simulink model "SyDdemo"  (Simulink 7.0)
settings: Solver=FixedStepDiscrete  StartTime=0.0  StopTime=10.0  FixedStep=0.01  RelTol=1e-3
model workspace (MAT data in the model file):
  air_esti_error : Simulink.Signal (value not decoded)
  atmo_pressure : Simulink.Signal (value not decoded)
  battery_volt : Simulink.Signal (value not decoded)
  control_pwm : Simulink.Signal (value not decoded)
  control_volt : Simulink.Signal (value not decoded)
  controller_error : Simulink.Signal (value not decoded)
  controller_error_lim : Simulink.Signal (value not decoded)
  controller_r0_out : Simulink.Signal (value not decoded)
  controller_r1_out : Simulink.Signal (value not decoded)
  controller_r2_out : Simulink.Signal (value not decoded)
  controller_r3_out : Simulink.Signal (value not decoded)
  controller_r_out : Simulink.Signal (value not decoded)
  controller_s0_out : Simulink.Signal (value not decoded)
  controller_s1_out : Simulink.Signal (value not decoded)
  controller_s2_out : Simulink.Signal (value not decoded)
  controller_s_out : Simulink.Signal (value not decoded)
  controller_s_out_prev : Simulink.Signal (value not decoded)
  controller_t0_out : Simulink.Signal (value not decoded)
  controller_t1_out : Simulink.Signal (value not decoded)
  controller_t2_out : Simulink.Signal (value not decoded)
  controller_t_out : Simulink.Signal (value not decoded)
  coolant_temp : Simulink.Signal (value not decoded)
  diag_control_ena : Simulink.Signal (value not decoded)
  diag_sp_ena : Simulink.Signal (value not decoded)
  engine_speed : Simulink.Signal (value not decoded)
  engine_status : Simulink.Signal (value not decoded)
  friction_comp_volt : Simulink.Signal (value not decoded)
  global_control_error : Simulink.Signal (value not decoded)
  pos_dmnd_raw : Simulink.Signal (value not decoded)
  pos_feedback : Simulink.Signal (value not decoded)
  pos_offset : Simulink.Signal (value not decoded)
  pos_relative : Simulink.Signal (value not decoded)
  pos_setpoint : Simulink.Signal (value not decoded)
  COR_COOLANT_TEMP_XDAT : Simulink.Parameter (value not decoded)
  COR_COOLANT_TEMP_YDAT : Simulink.Parameter (value not decoded)
  COR_ATMO_PRESSURE_XDAT : Simulink.Parameter (value not decoded)
  COR_ATMO_PRESSURE_YDAT : Simulink.Parameter (value not decoded)
  COR_AIR_ESTI_ERROR_GAIN : Simulink.Parameter (value not decoded)
  CONTROLLER_T2 : Simulink.Parameter (value not decoded)
  CONTROLLER_T1 : Simulink.Parameter (value not decoded)
  CONTROLLER_T0 : Simulink.Parameter (value not decoded)
  CONTROLLER_SETPONT_SIGN : Simulink.Parameter (value not decoded)
  CONTROLLER_R3 : Simulink.Parameter (value not decoded)
  CONTROLLER_R2 : Simulink.Parameter (value not decoded)
  CONTROLLER_R1 : Simulink.Parameter (value not decoded)
  CONTROLLER_SIGN : Simulink.Parameter (value not decoded)
  CONTROLLER_R0 : Simulink.Parameter (value not decoded)
  CONTROLLER_S2 : Simulink.Parameter (value not decoded)
  CONTROLLER_S1 : Simulink.Parameter (value not decoded)
  CONTROLLER_S0 : Simulink.Parameter (value not decoded)
  CONTROLLER_ERROR_LIM : Simulink.Parameter (value not decoded)
  FRICTION_DYN_COMP_VOLT : Simulink.Parameter (value not decoded)
  FRICTION_COMP_THR : Simulink.Parameter (value not decoded)
  FRICTION_STATIC_COMP_VOLT : Simulink.Parameter (value not decoded)
  BATTERY_COR_ENA : Simulink.Parameter (value not decoded)
  BATTERY_COR_PWM : Simulink.Parameter (value not decoded)
  DIAG_ENGINE_SPEED_MAX : Simulink.Parameter (value not decoded)
  DIAG_AIR_ESTI_ERROR_MAX : Simulink.Parameter (value not decoded)
  DIAG_POS_DMND_RAW_MIN : Simulink.Parameter (value not decoded)
  RUNNING : Simulink.Parameter (value not decoded)
  DIAG_ENGINE_SPEED_MIN : Simulink.Parameter (value not decoded)
  DIAG_COOLANT_TEMP_MAX : Simulink.Parameter (value not decoded)
  DIAG_COOLANT_TEMP_MIN : Simulink.Parameter (value not decoded)
  DIAG_ATMO_PRESSURE_MAX : Simulink.Parameter (value not decoded)
  C_GAIN : Simulink.Parameter (value not decoded)
libraries: simulink x12
settings Model: Creator=Aurelien PROST  LastModifiedBy=LeanPulse
settings Model: ModifiedHistory=|
  | <syd:extradata xmlns:syd="http://www.leanpulse.com/schemas/syd/2011/core" owner="LeanPulse" ref="SPEC0001" title="SyD Demo Document"><syd:version author="Aurélien PROST" date="10 Jan 2011" num="1.0" opt1="a.prost@leanpulse.com" opt2="">This specification has been created for demo purpose in order to highlight the main features of the LeanPulse System Document Generator (SyD).
  | 
  | (c) LeanPulse 2011</syd:version><syd:version author="Aurélien PROST" date="11 Jan 2011" num="2.0" opt1="a.prost@leanpulse.com" opt2="">You can edit this history by using the document metadata editor available in the file menu of your model.</syd:version></syd:extradata>
settings OptimizationCC: InitFltsAndDblsToZero=off
settings DebuggingCC: StrictBusMsg=ErrorLevel1

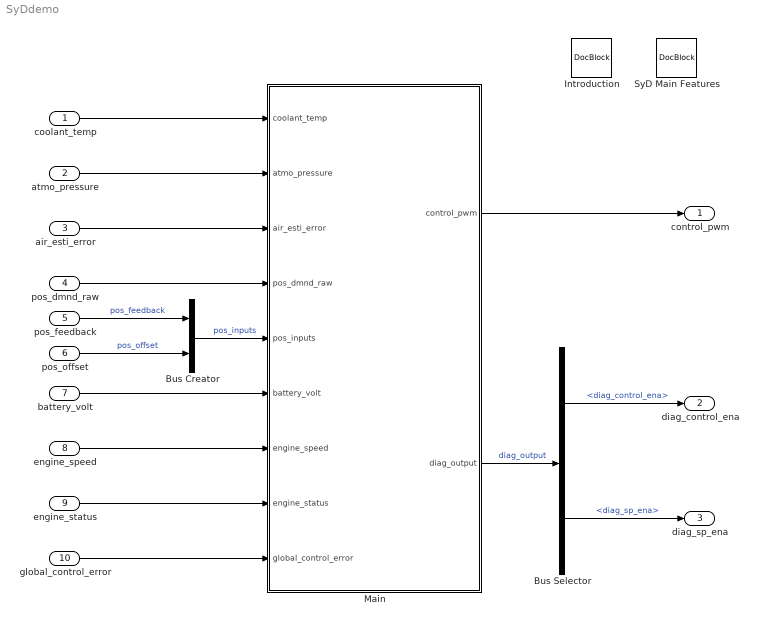
== system SyDdemo ==
  coolant_temp : Inport
  atmo_pressure : Inport  Port=2
  air_esti_error : Inport  Port=3
  pos_dmnd_raw : Inport  Port=4
  pos_feedback : Inport  Port=5
  pos_offset : Inport  Port=6
  battery_volt : Inport  Port=7
  engine_speed : Inport  Port=8
  engine_status : Inport  Port=9
  global_control_error : Inport  Port=10
  Bus Creator : BusCreator  Inputs=2
  Bus Selector : BusSelector  OutputSignals=diag_control_ena,diag_sp_ena
  Introduction : simulink/Model-Wide Utilities/DocBlock  SystemSampleTime=-1  DocumentType=RTF
  Main : Subsystem
  SyD Main Features : simulink/Model-Wide Utilities/DocBlock  SystemSampleTime=-1  DocumentType=RTF
  control_pwm : Outport
  diag_control_ena : Outport  Port=2
  diag_sp_ena : Outport  Port=3
  wires:
  coolant_temp/1 -> Main/1(coolant_temp)
  atmo_pressure/1 -> Main/2(atmo_pressure)
  air_esti_error/1 -> Main/3(air_esti_error)
  pos_dmnd_raw/1 -> Main/4(pos_dmnd_raw)
  battery_volt/1 -> Main/6(battery_volt)
  engine_speed/1 -> Main/7(engine_speed)
  engine_status/1 -> Main/8(engine_status)
  global_control_error/1 -> Main/9(global_control_error)
  Main/1(control_pwm) -> control_pwm/1
  Bus Selector/1 -> diag_control_ena/1  "<diag_control_ena>"
  Bus Selector/2 -> diag_sp_ena/1  "<diag_sp_ena>"
  pos_offset/1 -> Bus Creator/2  "pos_offset"
  pos_feedback/1 -> Bus Creator/1  "pos_feedback"
  Bus Creator/1 -> Main/5(pos_inputs)  "pos_inputs"
  Main/2(diag_output) -> Bus Selector/1  "diag_output"

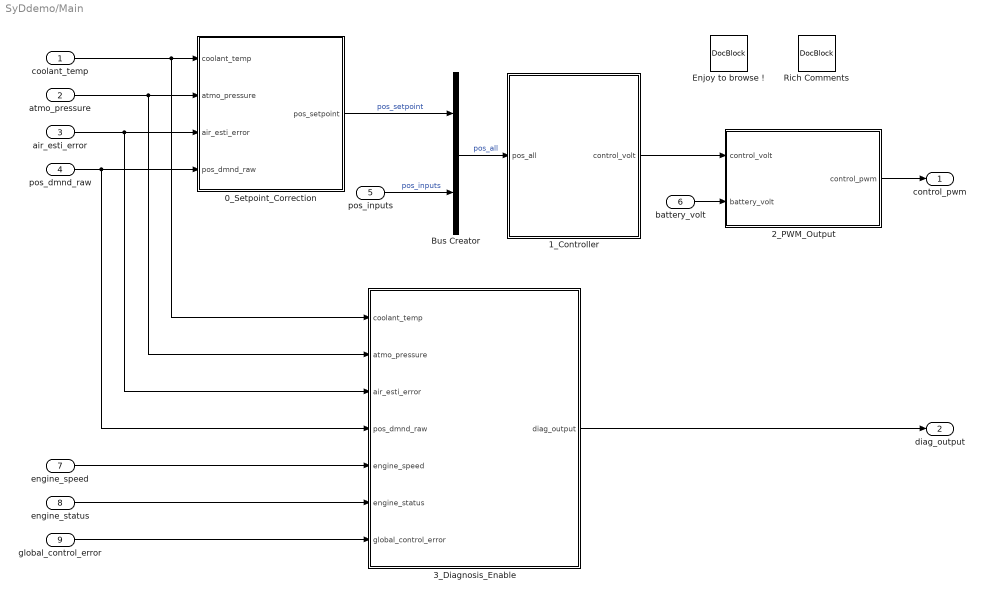
== system SyDdemo/Main ==
  coolant_temp : Inport
  atmo_pressure : Inport  Port=2
  air_esti_error : Inport  Port=3
  pos_dmnd_raw : Inport  Port=4
  pos_inputs : Inport  Port=5
  battery_volt : Inport  Port=6
  engine_speed : Inport  Port=7
  engine_status : Inport  Port=8
  global_control_error : Inport  Port=9
  0_Setpoint_Correction : Subsystem  Description="This comment has been written in the description field of the SubSystem \"0_Setpoint_Correction\"."
  1_Controller : Subsystem
  2_PWM_Output : Subsystem
  3_Diagnosis_Enable : Subsystem
  Bus Creator : BusCreator  Inputs=2
  Enjoy to browse ! : simulink/Model-Wide Utilities/DocBlock  SystemSampleTime=-1  DocumentType=RTF
  Rich Comments : simulink/Model-Wide Utilities/DocBlock  SystemSampleTime=-1  DocumentType=RTF
  control_pwm : Outport
  diag_output : Outport  Port=2
  wires:
  1_Controller/1(control_volt) -> 2_PWM_Output/1(control_volt)
  coolant_temp/1 -> 0_Setpoint_Correction/1(coolant_temp), 3_Diagnosis_Enable/1(coolant_temp)
  atmo_pressure/1 -> 0_Setpoint_Correction/2(atmo_pressure), 3_Diagnosis_Enable/2(atmo_pressure)
  air_esti_error/1 -> 0_Setpoint_Correction/3(air_esti_error), 3_Diagnosis_Enable/3(air_esti_error)
  pos_dmnd_raw/1 -> 0_Setpoint_Correction/4(pos_dmnd_raw), 3_Diagnosis_Enable/4(pos_dmnd_raw)
  battery_volt/1 -> 2_PWM_Output/2(battery_volt)
  2_PWM_Output/1(control_pwm) -> control_pwm/1
  3_Diagnosis_Enable/1(diag_output) -> diag_output/1
  0_Setpoint_Correction/1(pos_setpoint) -> Bus Creator/1  "pos_setpoint"
  pos_inputs/1 -> Bus Creator/2  "pos_inputs"
  Bus Creator/1 -> 1_Controller/1(pos_all)  "pos_all"
  engine_speed/1 -> 3_Diagnosis_Enable/5(engine_speed)
  engine_status/1 -> 3_Diagnosis_Enable/6(engine_status)
  global_control_error/1 -> 3_Diagnosis_Enable/7(global_control_error)

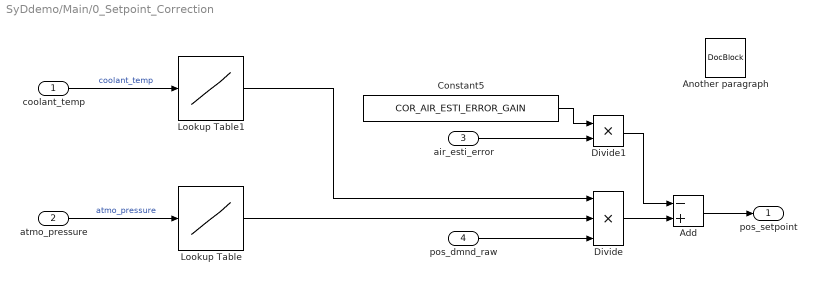
== system SyDdemo/Main/0_Setpoint_Correction ==
  coolant_temp : Inport
  atmo_pressure : Inport  Port=2
  air_esti_error : Inport  Port=3
  pos_dmnd_raw : Inport  Port=4
  Add : Sum  Inputs=-+
  Another paragraph : simulink/Model-Wide Utilities/DocBlock  SystemSampleTime=-1  DocumentType=RTF
  Constant5 : Constant  Value=COR_AIR_ESTI_ERROR_GAIN
  Divide : Product  Inputs=***
  Divide1 : Product  Inputs=**
  Lookup Table : Lookup  InputValues=COR_ATMO_PRESSURE_XDAT  Table=COR_ATMO_PRESSURE_YDAT
      settings: AttributesFormatString=Table: %<Table>\nInput Values: %<InputValues>
  Lookup Table1 : Lookup  InputValues=COR_COOLANT_TEMP_XDAT  Table=COR_COOLANT_TEMP_YDAT
      settings: AttributesFormatString=Table: %<Table>\nInput Values: %<InputValues>
  pos_setpoint : Outport
  wires:
  Lookup Table1/1 -> Divide/1
  Lookup Table/1 -> Divide/2
  pos_dmnd_raw/1 -> Divide/3
  air_esti_error/1 -> Divide1/2
  Constant5/1 -> Divide1/1
  Divide/1 -> Add/2
  Divide1/1 -> Add/1
  Add/1 -> pos_setpoint/1
  coolant_temp/1 -> Lookup Table1/1  "coolant_temp"
  atmo_pressure/1 -> Lookup Table/1  "atmo_pressure"

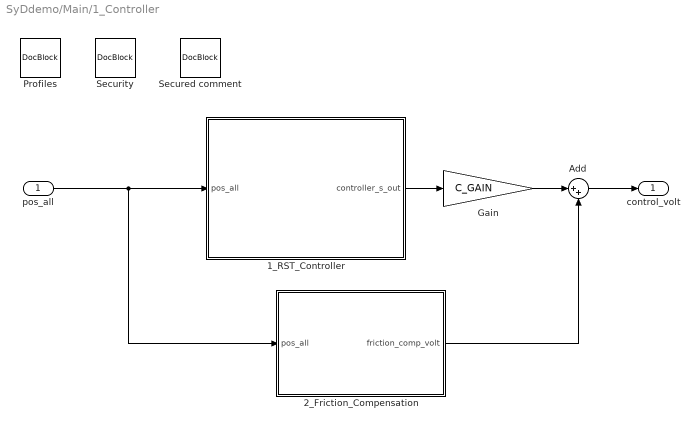
== system SyDdemo/Main/1_Controller ==
  pos_all : Inport
  1_RST_Controller : Subsystem
  2_Friction_Compensation : Subsystem
  Add : Sum  Inputs=|++
  Gain : Gain  Gain=C_GAIN
  Profiles : simulink/Model-Wide Utilities/DocBlock  SystemSampleTime=-1  DocumentType=RTF
  Secured comment : simulink/Model-Wide Utilities/DocBlock  Description="<syd:extradata xmlns:syd=\"http://www.leanpulse.com/schemas/syd/2011/core\" security=\"2\"/>"  SystemSampleTime=-1  DocumentType=RTF
  Security : simulink/Model-Wide Utilities/DocBlock  SystemSampleTime=-1  DocumentType=RTF
  control_volt : Outport
  wires:
  2_Friction_Compensation/1(friction_comp_volt) -> Add/2
  1_RST_Controller/1(controller_s_out) -> Gain/1
  Add/1 -> control_volt/1
  pos_all/1 -> 1_RST_Controller/1(pos_all), 2_Friction_Compensation/1(pos_all)
  Gain/1 -> Add/1

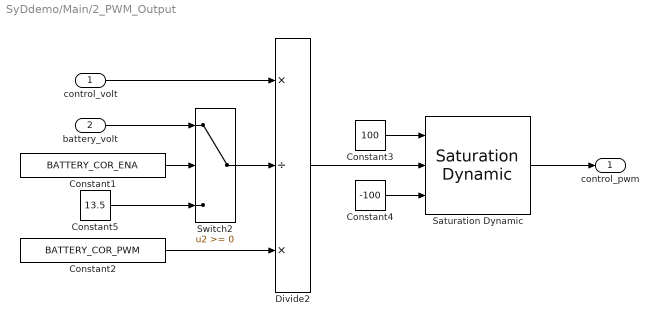
== system SyDdemo/Main/2_PWM_Output ==
  control_volt : Inport
  battery_volt : Inport  Port=2
  Constant1 : Constant  Value=BATTERY_COR_ENA
  Constant2 : Constant  Value=BATTERY_COR_PWM
  Constant3 : Constant  Value=100
  Constant4 : Constant  Value=-100
  Constant5 : Constant  Value=13.5
  Divide2 : Product  Inputs=*/*
  Saturation Dynamic : simulink/Discontinuities/Saturation Dynamic  SystemSampleTime=-1  OutMin=[]  OutMax=[]  OutDataTypeStr=Inherit: Same as second input  OutDataType=fixdt(1, 16)  OutScaling=2^-10  DoSatur=off
  Switch2 : Switch
  control_pwm : Outport
  wires:
  battery_volt/1 -> Switch2/1
  Constant1/1 -> Switch2/2
  Constant5/1 -> Switch2/3
  Switch2/1 -> Divide2/2
  control_volt/1 -> Divide2/1
  Constant2/1 -> Divide2/3
  Divide2/1 -> Saturation Dynamic/2
  Constant4/1 -> Saturation Dynamic/3
  Constant3/1 -> Saturation Dynamic/1
  Saturation Dynamic/1 -> control_pwm/1

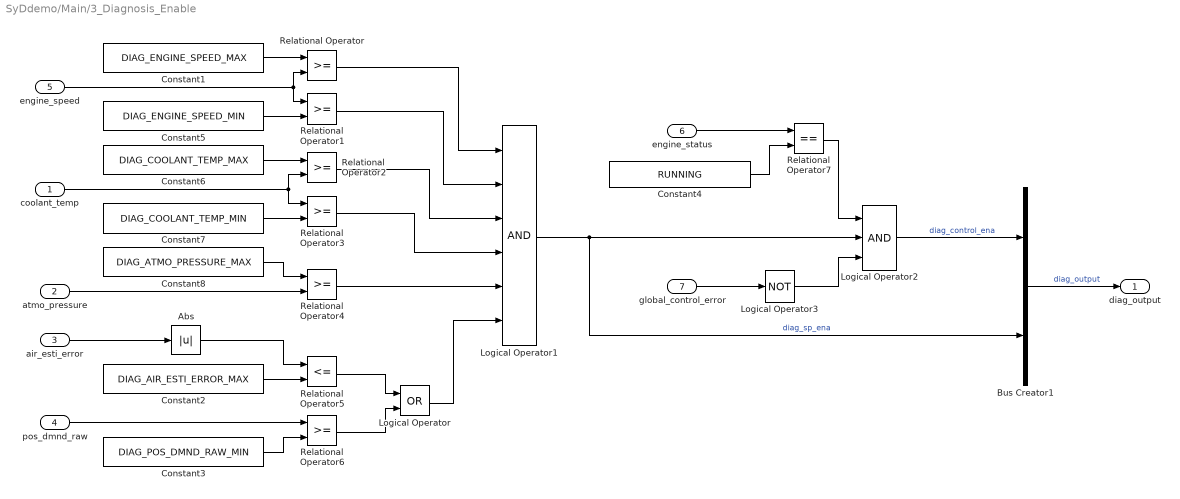
== system SyDdemo/Main/3_Diagnosis_Enable ==
  coolant_temp : Inport
  atmo_pressure : Inport  Port=2
  air_esti_error : Inport  Port=3
  pos_dmnd_raw : Inport  Port=4
  engine_speed : Inport  Port=5
  engine_status : Inport  Port=6
  global_control_error : Inport  Port=7
  Abs : Abs
  Bus Creator1 : BusCreator  Inputs=2
  Constant1 : Constant  Value=DIAG_ENGINE_SPEED_MAX
  Constant2 : Constant  Value=DIAG_AIR_ESTI_ERROR_MAX
  Constant3 : Constant  Value=DIAG_POS_DMND_RAW_MIN
  Constant4 : Constant  Value=RUNNING
  Constant5 : Constant  Value=DIAG_ENGINE_SPEED_MIN
  Constant6 : Constant  Value=DIAG_COOLANT_TEMP_MAX
  Constant7 : Constant  Value=DIAG_COOLANT_TEMP_MIN
  Constant8 : Constant  Value=DIAG_ATMO_PRESSURE_MAX
  Logical Operator : Logic  Operator=OR  AllPortsSameDT=off  OutDataTypeMode=boolean
  Logical Operator1 : Logic  Inputs=6  AllPortsSameDT=off  OutDataTypeMode=boolean
  Logical Operator2 : Logic  Inputs=3  AllPortsSameDT=off  OutDataTypeMode=boolean
  Logical Operator3 : Logic  Operator=NOT  AllPortsSameDT=off  OutDataTypeMode=boolean
  Relational Operator{,1..4,6} (6 alike) : RelationalOperator  LogicOutDataTypeMode=boolean
  Relational Operator5 : RelationalOperator  Operator=<=  LogicOutDataTypeMode=boolean
  Relational Operator7 : RelationalOperator  Operator===  LogicOutDataTypeMode=boolean
  diag_output : Outport
  wires:
  engine_speed/1 -> Relational Operator/2, Relational Operator1/1
  Constant1/1 -> Relational Operator/1
  Constant5/1 -> Relational Operator1/2
  coolant_temp/1 -> Relational Operator2/2, Relational Operator3/1
  Constant6/1 -> Relational Operator2/1
  Constant7/1 -> Relational Operator3/2
  atmo_pressure/1 -> Relational Operator4/2
  Constant8/1 -> Relational Operator4/1
  air_esti_error/1 -> Abs/1
  Abs/1 -> Relational Operator5/1
  Constant2/1 -> Relational Operator5/2
  pos_dmnd_raw/1 -> Relational Operator6/1
  Constant3/1 -> Relational Operator6/2
  Relational Operator5/1 -> Logical Operator/1
  Relational Operator6/1 -> Logical Operator/2
  Relational Operator/1 -> Logical Operator1/1
  Relational Operator1/1 -> Logical Operator1/2
  Relational Operator2/1 -> Logical Operator1/3
  Relational Operator3/1 -> Logical Operator1/4
  Relational Operator4/1 -> Logical Operator1/5
  Logical Operator/1 -> Logical Operator1/6
  Logical Operator1/1 -> Logical Operator2/2, Bus Creator1/2  "diag_sp_ena"
  Constant4/1 -> Relational Operator7/2
  engine_status/1 -> Relational Operator7/1
  Relational Operator7/1 -> Logical Operator2/1
  global_control_error/1 -> Logical Operator3/1
  Logical Operator3/1 -> Logical Operator2/3
  Logical Operator2/1 -> Bus Creator1/1  "diag_control_ena"
  Bus Creator1/1 -> diag_output/1  "diag_output"

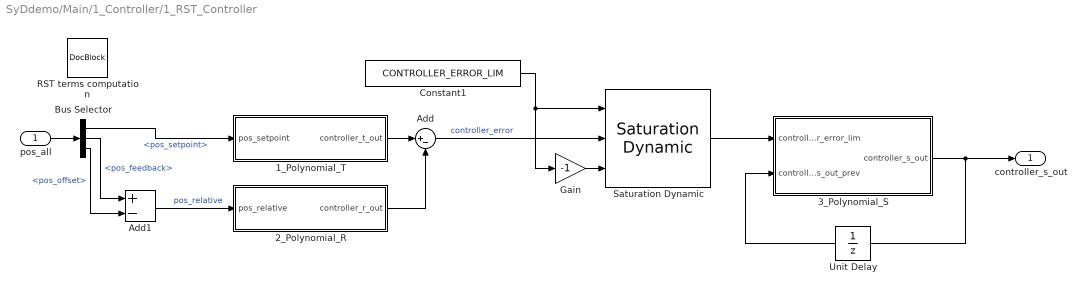
== system SyDdemo/Main/1_Controller/1_RST_Controller ==
  pos_all : Inport
  1_Polynomial_T : Subsystem
  2_Polynomial_R : Subsystem
  3_Polynomial_S : Subsystem
  Add : Sum  Inputs=|+-
  Add1 : Sum  Inputs=+-
  Bus Selector : BusSelector  OutputSignals=pos_setpoint,pos_inputs.pos_feedback,pos_inputs.pos_offset
  Constant1 : Constant  Value=CONTROLLER_ERROR_LIM
  Gain : Gain  Gain=-1
  RST terms computation : simulink/Model-Wide Utilities/DocBlock  SystemSampleTime=-1  DocumentType=RTF
  Saturation Dynamic : simulink/Discontinuities/Saturation Dynamic  SystemSampleTime=-1  OutMin=[]  OutMax=[]  OutDataTypeStr=Inherit: Same as second input  OutDataType=fixdt(1, 16)  OutScaling=2^-10  DoSatur=off
  Unit Delay : UnitDelay  SampleTime=-1
      settings: AttributesFormatString=Init=%<X0>
  controller_s_out : Outport
  wires:
  Add1/1 -> 2_Polynomial_R/1(pos_relative)  "pos_relative"
  1_Polynomial_T/1(controller_t_out) -> Add/1
  2_Polynomial_R/1(controller_r_out) -> Add/2
  Add/1 -> Saturation Dynamic/2  "controller_error"
  Constant1/1 -> Saturation Dynamic/1, Gain/1
  Gain/1 -> Saturation Dynamic/3
  Saturation Dynamic/1 -> 3_Polynomial_S/1(controller_error_lim)
  Unit Delay/1 -> 3_Polynomial_S/2(controller_s_out_prev)
  3_Polynomial_S/1(controller_s_out) -> controller_s_out/1, Unit Delay/1
  pos_all/1 -> Bus Selector/1
  Bus Selector/1 -> 1_Polynomial_T/1(pos_setpoint)  "<pos_setpoint>"
  Bus Selector/2 -> Add1/1  "<pos_feedback>"
  Bus Selector/3 -> Add1/2  "<pos_offset>"

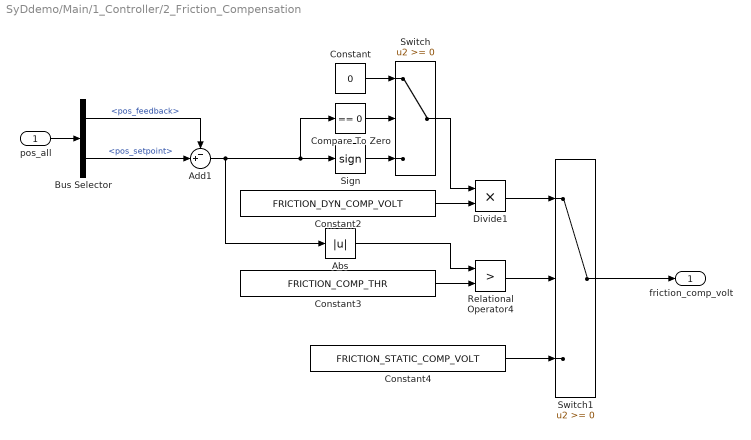
== system SyDdemo/Main/1_Controller/2_Friction_Compensation ==
  pos_all : Inport
  Abs : Abs
  Add1 : Sum  Inputs=-+|
  Bus Selector : BusSelector  OutputSignals=pos_inputs.pos_feedback,pos_setpoint
  Compare To Zero : simulink/Logic and Bit Operations/Compare To Zero  SystemSampleTime=-1  relop===  LogicOutDataTypeMode=boolean
  Constant : Constant  Value=0
  Constant2 : Constant  Value=FRICTION_DYN_COMP_VOLT
  Constant3 : Constant  Value=FRICTION_COMP_THR
  Constant4 : Constant  Value=FRICTION_STATIC_COMP_VOLT
  Divide1 : Product  Inputs=**
  Relational Operator4 : RelationalOperator  Operator=>  LogicOutDataTypeMode=boolean
  Sign : Signum
  Switch : Switch
  Switch1 : Switch
  friction_comp_volt : Outport
  wires:
  Add1/1 -> Sign/1, Compare To Zero/1, Abs/1
  Compare To Zero/1 -> Switch/2
  Sign/1 -> Switch/3
  Constant/1 -> Switch/1
  Constant2/1 -> Divide1/2
  Switch/1 -> Divide1/1
  Constant3/1 -> Relational Operator4/2
  Abs/1 -> Relational Operator4/1
  Relational Operator4/1 -> Switch1/2
  Divide1/1 -> Switch1/1
  Constant4/1 -> Switch1/3
  Switch1/1 -> friction_comp_volt/1
  pos_all/1 -> Bus Selector/1
  Bus Selector/1 -> Add1/1  "<pos_feedback>"
  Bus Selector/2 -> Add1/2  "<pos_setpoint>"

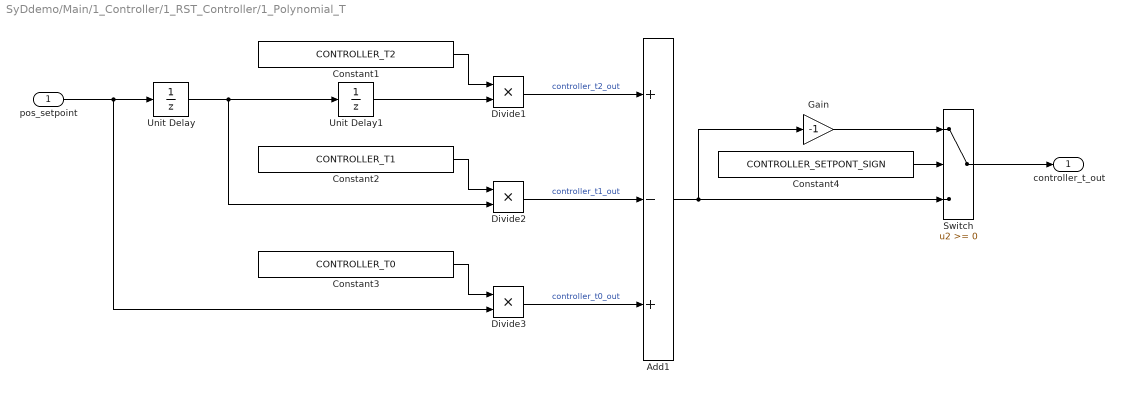
== system SyDdemo/Main/1_Controller/1_RST_Controller/1_Polynomial_T ==
  pos_setpoint : Inport
  Add1 : Sum  Inputs=+-+
  Constant1 : Constant  Value=CONTROLLER_T2
  Constant2 : Constant  Value=CONTROLLER_T1
  Constant3 : Constant  Value=CONTROLLER_T0
  Constant4 : Constant  Value=CONTROLLER_SETPONT_SIGN
  Divide1 : Product  Inputs=**
  Divide2 : Product  Inputs=**
  Divide3 : Product  Inputs=**
  Gain : Gain  Gain=-1
  Switch : Switch
  Unit Delay : UnitDelay  SampleTime=-1
      settings: AttributesFormatString=Init=%<X0>
  Unit Delay1 : UnitDelay  SampleTime=-1
      settings: AttributesFormatString=Init=%<X0>
  controller_t_out : Outport
  wires:
  Constant1/1 -> Divide1/1
  Unit Delay1/1 -> Divide1/2
  Constant2/1 -> Divide2/1
  Constant3/1 -> Divide3/1
  Unit Delay/1 -> Unit Delay1/1, Divide2/2
  pos_setpoint/1 -> Unit Delay/1, Divide3/2
  Divide2/1 -> Add1/2  "controller_t1_out"
  Divide1/1 -> Add1/1  "controller_t2_out"
  Divide3/1 -> Add1/3  "controller_t0_out"
  Switch/1 -> controller_t_out/1
  Add1/1 -> Switch/3, Gain/1
  Constant4/1 -> Switch/2
  Gain/1 -> Switch/1

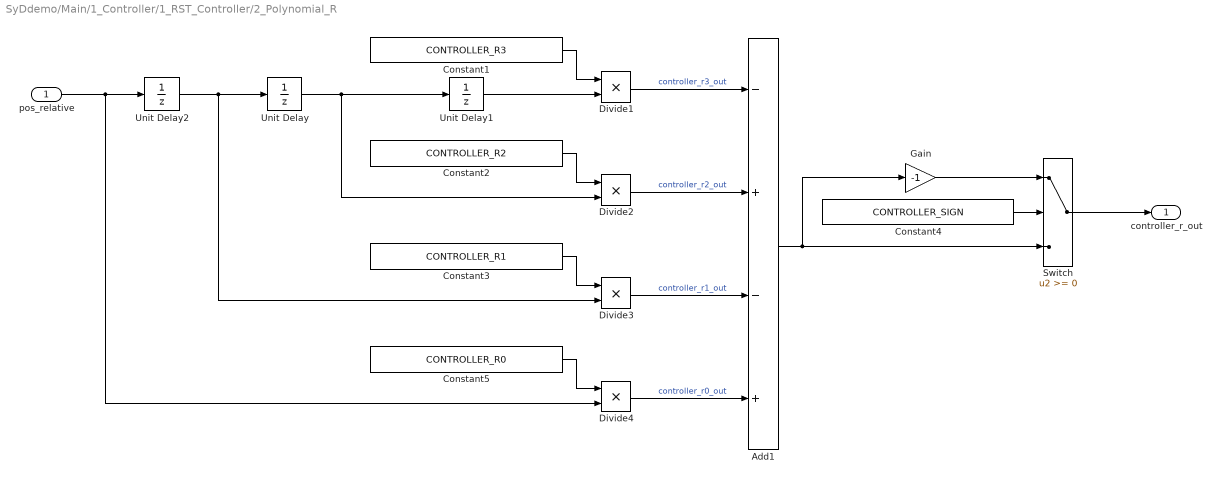
== system SyDdemo/Main/1_Controller/1_RST_Controller/2_Polynomial_R ==
  pos_relative : Inport
  Add1 : Sum  Inputs=-+-+
  Constant1 : Constant  Value=CONTROLLER_R3
  Constant2 : Constant  Value=CONTROLLER_R2
  Constant3 : Constant  Value=CONTROLLER_R1
  Constant4 : Constant  Value=CONTROLLER_SIGN
  Constant5 : Constant  Value=CONTROLLER_R0
  Divide{1..4} (4 alike) : Product  Inputs=**
  Gain : Gain  Gain=-1
  Switch : Switch
  Unit Delay : UnitDelay  SampleTime=-1
      settings: AttributesFormatString=Init=%<X0>
  Unit Delay1 : UnitDelay  SampleTime=-1
      settings: AttributesFormatString=Init=%<X0>
  Unit Delay2 : UnitDelay  SampleTime=-1
      settings: AttributesFormatString=Init=%<X0>
  controller_r_out : Outport
  wires:
  Gain/1 -> Switch/1
  Constant4/1 -> Switch/2
  Add1/1 -> Gain/1, Switch/3
  Switch/1 -> controller_r_out/1
  Divide3/1 -> Add1/3  "controller_r1_out"
  Divide1/1 -> Add1/1  "controller_r3_out"
  Divide2/1 -> Add1/2  "controller_r2_out"
  pos_relative/1 -> Unit Delay2/1, Divide4/2
  Unit Delay/1 -> Divide2/2, Unit Delay1/1
  Constant3/1 -> Divide3/1
  Constant2/1 -> Divide2/1
  Unit Delay1/1 -> Divide1/2
  Constant1/1 -> Divide1/1
  Divide4/1 -> Add1/4  "controller_r0_out"
  Constant5/1 -> Divide4/1
  Unit Delay2/1 -> Divide3/2, Unit Delay/1

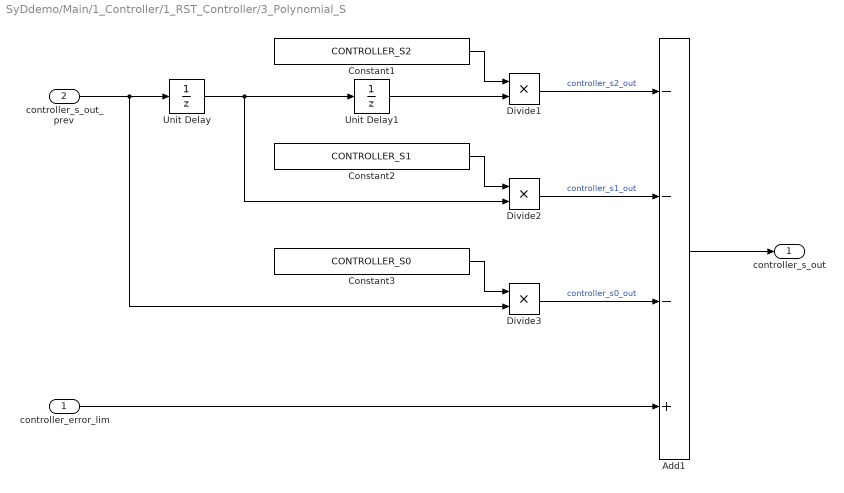
== system SyDdemo/Main/1_Controller/1_RST_Controller/3_Polynomial_S ==
  controller_error_lim : Inport
  controller_s_out_prev : Inport  Port=2
  Add1 : Sum  Inputs=---+
  Constant1 : Constant  Value=CONTROLLER_S2
  Constant2 : Constant  Value=CONTROLLER_S1
  Constant3 : Constant  Value=CONTROLLER_S0
  Divide1 : Product  Inputs=**
  Divide2 : Product  Inputs=**
  Divide3 : Product  Inputs=**
  Unit Delay : UnitDelay  SampleTime=-1
      settings: AttributesFormatString=Init=%<X0>
  Unit Delay1 : UnitDelay  SampleTime=-1
      settings: AttributesFormatString=Init=%<X0>
  controller_s_out : Outport
  wires:
  Constant1/1 -> Divide1/1
  Unit Delay1/1 -> Divide1/2
  Constant2/1 -> Divide2/1
  Constant3/1 -> Divide3/1
  Unit Delay/1 -> Unit Delay1/1, Divide2/2
  controller_s_out_prev/1 -> Unit Delay/1, Divide3/2
  Divide2/1 -> Add1/2  "controller_s1_out"
  Divide1/1 -> Add1/1  "controller_s2_out"
  Divide3/1 -> Add1/3  "controller_s0_out"
  controller_error_lim/1 -> Add1/4
  Add1/1 -> controller_s_out/1
